simulink model "tut3"  (Simulink 7.4)
settings: Solver=ode45  StartTime=0.0  StopTime=10.0  RelTol=1e-3
undefined symbols (not defined in the file): gcbh, pfb_coeff_gen_calc, xlBlockLoadCallback
libraries: xbsIndex_r4 x2537, casper_library_misc x93, casper_library_delays x38, xps_library x10, xbsReplacements_r4 x2, casper_library_pfbs x2, simulink x1, casper_library_ffts_twiddle x1
(part 15 of 44)

== system tut3/fft_wideband_real1/fft_direct/butterfly2_0/twiddle_general_dsp48e ==
  a : Inport
  b : Inport  Port=2
  sync : Inport  Port=3
  a_c_to_ri : casper_library_misc/c_to_ri  SystemSampleTime=-1  n_bits=input_bit_width  bin_pt=input_bit_width-1
  a_delay : xbsIndex_r4/Delay  en=off  latency=bram_latency + 4 + conv_latency  dbl_ovrd=off  reg_retiming=off  block_type=delay
      settings: block_version=11.4
  b_c_to_ri : casper_library_misc/c_to_ri  SystemSampleTime=-1  n_bits=input_bit_width  bin_pt=input_bit_width-1
  b_delay : xbsIndex_r4/Delay  en=off  latency=bram_latency  dbl_ovrd=off  reg_retiming=on  block_type=delay
      settings: block_version=11.4
  cmult : Subsystem  AncestorBlock=casper_library_multipliers/cmult_dsp48e  mask(cmult_dsp48e){n_bits_a=input_bit_width, bin_pt_a=input_bit_width - 1, n_bits_b=coeff_bit_width, bin_pt_b=coeff_bit_width - 2, conjugated=off, full_precision=off, n_bits_c=input_bit_width + 4, bin_pt_c=input_bit_width + 1, quantization=Round  (unbiased: Even Values), overflow=Wrap, cast_latency=conv_latency}
      mask prompts: n_bits_a "Bit width 'a'"; bin_pt_a "Binary point 'a'"; n_bits_b "Bit width 'b'"; bin_pt_b "Binary point 'b'"; conjugated "Conjugate"; full_precision "Full precision"; n_bits_c "Bit width out"; bin_pt_c "Binary point out"; cast_latency "Cast Latency"
      mask help:
      | Multiplies two complex numbers using 4 DSP48Es.
      | Conjugation is optional.
      |
      | Re{a} and Im{a} can be up to 25 bits.
      | Re{b} and Im{b} can be up to 18 bits.
      mask init: same as tut3/fft_wideband_real1/fft_direct/butterfly1_0/twiddle_general_dsp48e/cmult
      settings: AttributesFormatString=|
      | 18_17 * 18_16 ==> 22_19
      | Latency=5
      mask StyleString: edit,edit,edit,edit,checkbox,checkbox,edit,edit,popup(Truncate|Round  (unbiased: +/- Inf)|Round  (unbiased: Even Values)),popup(Wrap|Saturate|Flag as error),edit
      mask CallbackString:
      | |||||full_precision = get_param(gcb, 'full_precision');
      | 
      | if strcmp(full_precision, 'on'),
      |     not_full_precision = 'off';
      | else
      |     not_full_precision = 'on';
      | end
      | 
      | set_param_state(gcb, 'n_bits_c', not_full_precision);
      | set_param_state(gcb, 'bin_pt_c', not_full_precision);
      | set_param_state(gcb, 'quantization', not_full_precision);
      | set_param_state(gcb, 'overflow', not_full_precision);
      | set_param_state(gcb, 'cast_latency', not_full_precision);|||||
      mask TabNameString: Basic,Basic,Basic,Basic,Basic,Output Type,Output Type,Output Type,Output Type,Output Type,Output Type
  coeff_c_to_ri : casper_library_misc/c_to_ri  SystemSampleTime=-1  n_bits=coeff_bit_width  bin_pt=coeff_bit_width-2
      settings: AttributesFormatString=18_16 r/i
  coeff_gen : Subsystem  AncestorBlock=casper_library_ffts_twiddle_coeff_gen/coeff_gen  mask(coeff_gen){Coeffs=[1+0i       0.99999882345170187925020854891045-0.0015339801862847655001403923691328i       0.99999529380957619117964441102231-0.0030679567629659761432425746363606i       0.99998941108192840321322591989883-0.0046019261204485704952471536444136i       0.99998117528260110908888691483298-0.0061358846491544752690949771078976i        0.99997058643097413987987920336309-0.007669828739531097012982474581122i       0.99995764455196389786095778617891-0.0092037547820598194364682953505508i        0.99994234967602391161989316969994-0.010737659167264490545079524963512i        0.99992470183914450299056397852837-0.012271538285719925387429185548172i        0.99990470108285289807525941796484-0.013805388528060390587737238377031i        0.99988234745421256111086449891445-0.015339206284988100154054180279672i        0.99985764100582386060267481298069-0.016872987947281710419122902067102i        0.99983058179582340319058175737155-0.018406729905804820185410974886508i         0.99980116988788425569367745993077-0.01994042855151444137984384497031i        0.99976940535121527897644000404398-0.021474080275469507872454499874948i        0.99973528826056168306024574121693-0.023007681468839368682521850928424i        0.99969881869620424996725205346593-0.024541228522912288123603019585062i        0.99965999674395922269809489080217-0.026074717829103900845755248383284i        0.99961882249517863829879615877871-0.027608145778965739736143802929291i        0.99957529604674921763773909333395-0.029141508764193722186286450437365i        0.99952941750109314256178549840115-0.030674803176636625950957082409332i        0.99948118696616694567325112075196-0.032208025408304585823771049035713i         0.99943060455546173237451057502767-0.03374117185137757990442963773603i         0.9993776703880028478010899561923-0.035274238898213947090987119281635i        0.99932238458834954375475945198559-0.036807222941358831713731802892653i        0.99926474728659442359202103034477-0.038340120373552694088559178453579i        0.99920475861836388631331828946713-0.039872927587739810662004202868047i        0.99914241872481690531770937013789-0.041405640977076739461804066877448i        0.99907772775264536146977434327709-0.042938256934940820241930481415693i        0.99901068585407337696580043484573-0.044470771854938667688728060056746i        0.99894129318685687124457217578311-0.046003182130914622993689278018792i        0.99886954991428356098737140200683-0.047535484156959302570744085869592i        0.99879545620517240500646494183457-0.049067674327418014934565348994511i        0.99871901223387293811128984089009-0.050599749036899281662282845672962i        0.99864021818026527110845336210332-0.052131704680283323660727745618715i        0.99855907422975931364561574810068-0.053663537652730519678101472891285i        0.99847558057329477421149022120517-0.055195244349689941143477511786841i        0.99838973740734016093512082079542-0.056726821166907748228425134584541i        0.99830154493389289260818486582139-0.058258264500435752442086823066347i        0.99821100336047818846196832964779-0.059789570746639868203953227521197i        0.99811811290014917918966830256977-0.061320736302208578294425933563616i        0.99802287377148624081257821671898-0.062851757564161406244274132859573i        0.99792528619859599547936568342266-0.064382630929857465051924236831837i        0.99782535041111164453297988075064-0.065913352797003804672826277055719i           0.9977230666441916362430220033275-0.0674439195636640509423642697584i        0.99761843513851955478344279981684-0.068974327628266746126328712307441i        0.99751145614030345409872779782745-0.070504573389613856004842773472774i        0.99740212990127530279238499133498-0.072034653246889332467439714946522i        0.99729045667869020697082760307239-0.073564563599667426307959772202594i        0.99717643673532618819876915949862-0.075094300847921305330778807274328i        0.99706007033948296225389640312642-0.076623861392031492045795459944202i ...(+76860 chars)], coeff_bit_width=coeff_bit_width, StepPeriod=0, bram_latency=bram_latency, coeffs_bram=on}
      mask prompts: Coeffs "Coefficients: (0 to 2^(FFTSize-1)-1)"; coeff_bit_width "Coefficient Bit Width:"; StepPeriod "Step Period: (2^?)"; bram_latency "Latency:"; coeffs_bram "Store coefficients in BRAM"
      mask help: Generates a sequence of complex coefficients
      mask init:
      | coeff_gen_init( gcb, ...
      |     'Coeffs', Coeffs, ...
      |     'coeff_bit_width', coeff_bit_width, ...
      |     'StepPeriod', StepPeriod, ...
      |     'bram_latency', bram_latency, ...
      |     'coeffs_bram', coeffs_bram);
      settings: AttributesFormatString=1024 @ (18,16)
      mask CallbackString:
      | Coeffs = eval(get_param(gcb, 'Coeffs'));
      | if( length(Coeffs) == 1 ),
      |     set_param(gcb, 'MaskVisibilities',{'on','on','off','off','off'});
      | else
      |     set_param(gcb, 'MaskVisibilities',{'on','on','on','on','on'});
      | end||||
  sync_delay : xbsIndex_r4/Delay  en=off  latency=bram_latency + 4 + conv_latency  dbl_ovrd=off  reg_retiming=off  block_type=delay
      settings: block_version=11.4
  a_re : Outport
  a_im : Outport  Port=2
  bw_re : Outport  Port=3
  bw_im : Outport  Port=4
  sync_out : Outport  Port=5
  wires:
  a/1 -> a_delay/1
  a_delay/1 -> a_c_to_ri/1
  a_c_to_ri/1 -> a_re/1
  a_c_to_ri/2 -> a_im/1
  b/1 -> b_delay/1
  b_delay/1 -> b_c_to_ri/1
  b_c_to_ri/1 -> cmult/1(a_re)
  b_c_to_ri/2 -> cmult/2(a_im)
  sync/1 -> coeff_gen/1(rst), sync_delay/1
  coeff_gen/1(w) -> coeff_c_to_ri/1
  coeff_c_to_ri/1 -> cmult/3(b_re)
  coeff_c_to_ri/2 -> cmult/4(b_im)
  cmult/1(c_re) -> bw_re/1
  cmult/2(c_im) -> bw_im/1
  sync_delay/1 -> sync_out/1
